simulink model "HvacMdlAirFlowDistbnFrnt"  (R2022b)
settings: Solver=FixedStepDiscrete  StartTime=0.0  StopTime=10.0  FixedStep=0.2  RelTol=1e-3
(part 2 of 2)

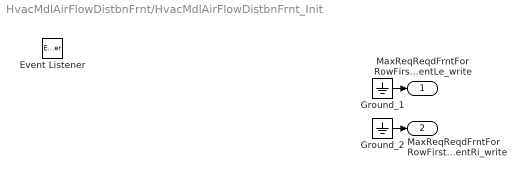
== system HvacMdlAirFlowDistbnFrnt/HvacMdlAirFlowDistbnFrnt_Init ==
  Event Listener : EventListener  EventName=reset
  Ground_1 : Ground
  Ground_2 : Ground
  MaxReqReqdFrntForRowFirstAtVent_MaxReqReqdFrntForRowFirstAtVentLe_write : Outport
  MaxReqReqdFrntForRowFirstAtVent_MaxReqReqdFrntForRowFirstAtVentRi_write : Outport  Port=2
  wires:
  Ground_1/1 -> MaxReqReqdFrntForRowFirstAtVent_MaxReqReqdFrntForRowFirstAtVentLe_write/1
  Ground_2/1 -> MaxReqReqdFrntForRowFirstAtVent_MaxReqReqdFrntForRowFirstAtVentRi_write/1

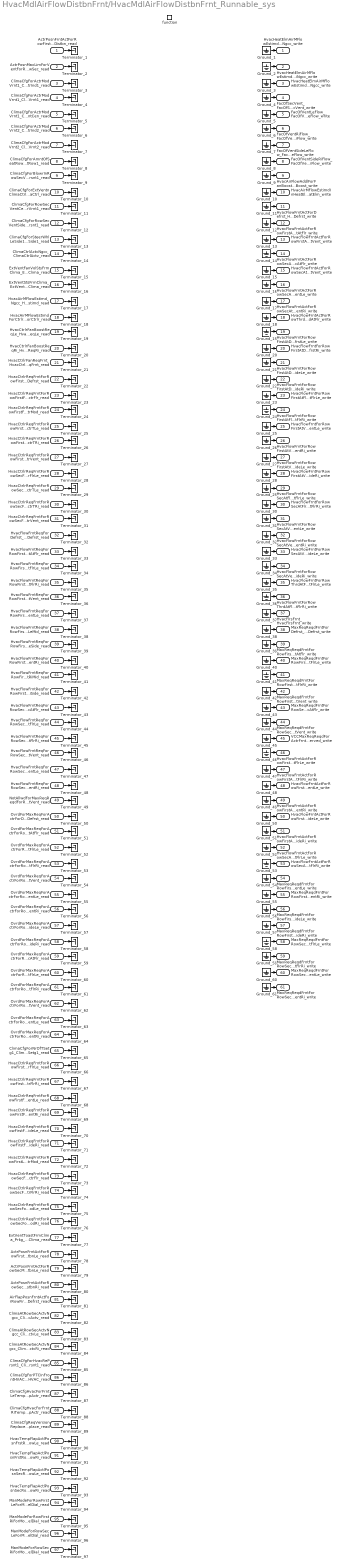
== system HvacMdlAirFlowDistbnFrnt/HvacMdlAirFlowDistbnFrnt_Runnable_sys ==
  ActrPosnFrntActForRowFirstModDistbn_ActrPosnFrntActForRowFirstModDistbn_read : Inport
  ActrPosnMaxLimForVentForRowSec_ActrPosnMaxLimForVentForRowSec_read : Inport  Port=2
  ClimaCfgForActrModVrnt1_ClimaCfgForActrInd1_read : Inport  Port=3
  ClimaCfgForActrModVrnt1_ClimaCfgForActrModVrnt1_read : Inport  Port=4
  ClimaCfgForActrModVrnt1_ClimaCfgForActrModVrntCen_read : Inport  Port=5
  ClimaCfgForActrModVrnt2_ClimaCfgForActrInd2_read : Inport  Port=6
  ClimaCfgForActrModVrnt2_ClimaCfgForActrModVrnt2_read : Inport  Port=7
  ClimaCfgForAmntOfSeatRow1_ClimaCfgForAmntOfSeatRow1_read : Inport  Port=8
  ClimaCfgForBlowrInRowSecVentSidePrsnt1_ClimaCfgForBlowrInRowSecVentSidePrsnt1_read : Inport  Port=9
  ClimaCfgForExtVentnClimaCtrl_ClimaCfgForExtVentnClimaCtrl_read : Inport  Port=10
  ClimaCfgForRowSecVentCentrVrnt1_ClimaCfgForRowSecVentCentrVrnt1_read : Inport  Port=11
  ClimaCfgForRowSecVentSidePrsnt1_ClimaCfgForRowSecVentSidePrsnt1_read : Inport  Port=12
  ClimaCfgForSteerWhlLeSide1_ClimaCfgForSteerWhlLeSide1_read : Inport  Port=13
  ClimaCtrlActvNgcc_ClimaCtrlActv_read : Inport  Port=14
  ExtVentFanVolStsFrmClima_ExtVentFanVolStsFrmClima_read : Inport  Port=15
  ExtVentStsFrmClima_ExtVentStsFrmClima_read : Inport  Port=16
  HvacAirMFlowEstimd_Ngcc_HvacAirMFlowEstimd_read : Inport  Port=17
  HvacAirMFlowEstimdForCtrlr_HvacAirMFlowEstimdForCtrlr_read : Inport  Port=18
  HvacCtrlrFanBoostReqLe_HvacCtrlrFanBoostReqLe_read : Inport  Port=19
  HvacCtrlrFanBoostReqRi_HvacCtrlrFanBoostReqRi_read : Inport  Port=20
  HvacCtrlrFanReqFrnt_HvacCtrlrFanReqFrnt_read : Inport  Port=21
  HvacCtrlrReqFrntForRowFirstForActrDefrst_HvacCtrlrReqFrntForRowFirstForActrDefrst_read : Inport  Port=22
  HvacCtrlrReqFrntForRowFirstForActrFlr_HvacCtrlrReqFrntForRowFirstForActrFlr_read : Inport  Port=23
  HvacCtrlrReqFrntForRowFirstForActrMod_HvacCtrlrReqFrntForRowFirstForActrMod_read : Inport  Port=24
  HvacCtrlrReqFrntForRowFirstForActrTLe_HvacCtrlrReqFrntForRowFirstForActrTLe_read : Inport  Port=25
  HvacCtrlrReqFrntForRowFirstForActrTRi_HvacCtrlrReqFrntForRowFirstForActrTRi_read : Inport  Port=26
  HvacCtrlrReqFrntForRowFirstForActrVent_HvacCtrlrReqFrntForRowFirstForActrVent_read : Inport  Port=27
  HvacCtrlrReqFrntForRowSecForActrFlr_HvacCtrlrReqFrntForRowSecForActrFlrLe_read : Inport  Port=28
  HvacCtrlrReqFrntForRowSecForActrTLe_HvacCtrlrReqFrntForRowSecForActrTLe_read : Inport  Port=29
  HvacCtrlrReqFrntForRowSecForActrTRi_HvacCtrlrReqFrntForRowSecForActrTRi_read : Inport  Port=30
  HvacCtrlrReqFrntForRowSecForActrVent_HvacCtrlrReqFrntForRowSecForActrVent_read : Inport  Port=31
  HvacFlowFrntReqForDefrst_HvacFlowFrntReqForDefrst_read : Inport  Port=32
  HvacFlowFrntReqForRowFirstAtFlr_HvacFlowFrntReqForRowFirstAtFlr_read : Inport  Port=33
  HvacFlowFrntReqForRowFirstAtFlr_HvacFlowFrntReqForRowFirstAtFlrLe_read : Inport  Port=34
  HvacFlowFrntReqForRowFirstAtFlr_HvacFlowFrntReqForRowFirstAtFlrRi_read : Inport  Port=35
  HvacFlowFrntReqForRowFirstAtVent_HvacFlowFrntReqForRowFirstAtVent_read : Inport  Port=36
  HvacFlowFrntReqForRowFirstAtVent_HvacFlowFrntReqForRowFirstAtVentLe_read : Inport  Port=37
  HvacFlowFrntReqForRowFirstAtVent_HvacFlowFrntReqForRowFirstAtVentLeMid_read : Inport  Port=38
  HvacFlowFrntReqForRowFirstAtVent_HvacFlowFrntReqForRowFirstAtVentLeSide_read : Inport  Port=39
  HvacFlowFrntReqForRowFirstAtVent_HvacFlowFrntReqForRowFirstAtVentRi_read : Inport  Port=40
  HvacFlowFrntReqForRowFirstAtVent_HvacFlowFrntReqForRowFirstAtVentRiMid_read : Inport  Port=41
  HvacFlowFrntReqForRowFirstAtVent_HvacFlowFrntReqForRowFirstAtVentRiSide_read : Inport  Port=42
  HvacFlowFrntReqForRowSecAtFlr_HvacFlowFrntReqForRowSecAtFlr_read : Inport  Port=43
  HvacFlowFrntReqForRowSecAtFlr_HvacFlowFrntReqForRowSecAtFlrLe_read : Inport  Port=44
  HvacFlowFrntReqForRowSecAtFlr_HvacFlowFrntReqForRowSecAtFlrRi_read : Inport  Port=45
  HvacFlowFrntReqForRowSecAtVent_HvacFlowFrntReqForRowSecAtVent_read : Inport  Port=46
  HvacFlowFrntReqForRowSecAtVent_HvacFlowFrntReqForRowSecAtVentLe_read : Inport  Port=47
  HvacFlowFrntReqForRowSecAtVent_HvacFlowFrntReqForRowSecAtVentRi_read : Inport  Port=48
  NotAllwdForMaxReqReqdForRowSecAtVent_NotAllwdForMaxReqReqdForRowSecAtVent_read : Inport  Port=49
  OvrdForMaxReqForActrForDefrst_OvrdForMaxReqForActrForDefrst_read : Inport  Port=50
  OvrdForMaxReqForActrForRowFirstAtFlr_OvrdForMaxReqForActrForRowFirstAtFlr_read : Inport  Port=51
  OvrdForMaxReqForActrForRowFirstAtFlr_OvrdForMaxReqForActrForRowFirstAtFlrLe_read : Inport  Port=52
  OvrdForMaxReqForActrForRowFirstAtFlr_OvrdForMaxReqForActrForRowFirstAtFlrRi_read : Inport  Port=53
  OvrdForMaxReqForActrForRowFirstAtVent_OvrdForMaxReqForActrForRowFirstAtVent_read : Inport  Port=54
  OvrdForMaxReqForActrForRowFirstAtVent_OvrdForMaxReqForActrForRowFirstAtVentLe_read : Inport  Port=55
  OvrdForMaxReqForActrForRowFirstAtVent_OvrdForMaxReqForActrForRowFirstAtVentRi_read : Inport  Port=56
  OvrdForMaxReqForActrForRowFirstAtVent_OvrdForMaxReqForActrForRowFirstAtVentSideLe_read : Inport  Port=57
  OvrdForMaxReqForActrForRowFirstAtVent_OvrdForMaxReqForActrForRowFirstAtVentSideRi_read : Inport  Port=58
  OvrdForMaxReqForActrForRowSecAtFlr_OvrdForMaxReqForActrForRowSecAtFlr_read : Inport  Port=59
  OvrdForMaxReqForActrForRowSecAtFlr_OvrdForMaxReqForActrForRowSecAtFlrLe_read : Inport  Port=60
  OvrdForMaxReqForActrForRowSecAtFlr_OvrdForMaxReqForActrForRowSecAtFlrRi_read : Inport  Port=61
  OvrdForMaxReqForActrForRowSecAtVent_OvrdForMaxReqForActrForRowSecAtVent_read : Inport  Port=62
  OvrdForMaxReqForActrForRowSecAtVent_OvrdForMaxReqForActrForRowSecAtVentLe_read : Inport  Port=63
  OvrdForMaxReqForActrForRowSecAtVent_OvrdForMaxReqForActrForRowSecAtVentRi_read : Inport  Port=64
  ClimaCfgForNrOfTSetg1_ClimaCfgForNrOfTSetg1_read : Inport  Port=65
  HvacCtrlrReqFrntForRowFirstForActrFlr_HvacCtrlrReqFrntForRowFirstForActrFlrLe_read : Inport  Port=66
  HvacCtrlrReqFrntForRowFirstForActrFlr_HvacCtrlrReqFrntForRowFirstForActrFlrRi_read : Inport  Port=67
  HvacCtrlrReqFrntForRowFirstForActrVent_HvacCtrlrReqFrntForRowFirstForActrVentLe_read : Inport  Port=68
  HvacCtrlrReqFrntForRowFirstForActrVent_HvacCtrlrReqFrntForRowFirstForActrVentRi_read : Inport  Port=69
  HvacCtrlrReqFrntForRowFirstForActrVent_HvacCtrlrReqFrntForRowFirstForActrVentSideLe_read : Inport  Port=70
  HvacCtrlrReqFrntForRowFirstForActrVent_HvacCtrlrReqFrntForRowFirstForActrVentSideRi_read : Inport  Port=71
  HvacCtrlrReqFrntForRowFirstLeForActrMod_HvacCtrlrReqFrntForRowFirstLeForActrMod_read : Inport  Port=72
  HvacCtrlrReqFrntForRowSecForActrFlr_HvacCtrlrReqFrntForRowSecForActrFlr_read : Inport  Port=73
  HvacCtrlrReqFrntForRowSecForActrFlr_HvacCtrlrReqFrntForRowSecForActrFlrRi_read : Inport  Port=74
  HvacCtrlrReqFrntForRowSecForActrModLe_HvacCtrlrReqFrntForRowSecForActrModLe_read : Inport  Port=75
  HvacCtrlrReqFrntForRowSecForActrModRi_HvacCtrlrReqFrntForRowSecForActrModRi_read : Inport  Port=76
  ExtVentToastFrmClima_Prkg_ExtVentToastFrmClima_read : Inport  Port=77
  ActrPosnFrntActForRowFirstModDistbnLe_ActrPosnFrntActForRowFirstModDistbnLe_read : Inport  Port=78
  ActrPosnFrntActForRowSecModDistbnLe_ActrPosnFrntActForRowSecModDistbnLe_read : Inport  Port=79
  ActrPosnFrntActForRowSecModDistbnRi_ActrPosnFrntActForRowSecModDistbnRi_read : Inport  Port=80
  AirFlapPosnFrntActForRowFirstForActrDefrst_AirFlapPosnFrntActForRowFirstForActrDefrst_read : Inport  Port=81
  ClimaAtRowSecActvNgcc_ClimaAtRowSecActv_read : Inport  Port=82
  ClimaAtRowSecActvNgcc_ClimaAtRowSecActvLe_read : Inport  Port=83
  ClimaAtRowSecActvNgcc_ClimaAtRowSecActvRi_read : Inport  Port=84
  ClimaCfgForHvacRePrsnt1_ClimaCfgForHvacRePrsnt1_read : Inport  Port=85
  ClimaCfgForPTCInFrontHVAC_ClimaCfgForPTCInFrontHVAC_read : Inport  Port=86
  ClimaCfgHvacForFrntLeTempActr_ClimaCfgHvacForFrntLeTempActr_read : Inport  Port=87
  ClimaCfgHvacForFrntRiTempActr_ClimaCfgHvacForFrntRiTempActr_read : Inport  Port=88
  ClimaCfgReqVersionReplace_ClimaCfgReqVersionReplace_read : Inport  Port=89
  HvacTempFlapActlPosnFrstRowLe_HvacTempFlapActlPosnFrstRowLe_read : Inport  Port=90
  HvacTempFlapActlPosnFrstRowRi_HvacTempFlapActlPosnFrstRowRi_read : Inport  Port=91
  HvacTempFlapActlPosnSecRowLe_HvacTempFlapActlPosnSecRowLe_read : Inport  Port=92
  HvacTempFlapActlPosnSecRowRi_HvacTempFlapActlPosnSecRowRi_read : Inport  Port=93
  ManModeForRowFirstLeForModelDial_ManModeForRowFirstLeForModelDial_read : Inport  Port=94
  ManModeForRowFirstRiForModelDial_ManModeForRowFirstRiForModelDial_read : Inport  Port=95
  ManModeForRowSecLeForModelDial_ManModeForRowSecLeForModelDial_read : Inport  Port=96
  ManModeForRowSecRiForModelDial_ManModeForRowSecRiForModelDial_read : Inport  Port=97
  function : TriggerPort  TriggerType=function-call  VariantControl=(inherit)  SampleTimeType=periodic  SampleTime=-1
  Ground_{1..61} (61 alike) : Ground
  Terminator_{1..97} (97 alike) : Terminator
  HvacHeatElmAirMFlowEstimdFirstLeNgcc_HvacHeatElmAirMFlowEstimdFirstLeNgcc_write : Outport
      settings: EnsureOutportIsVirtual=on
  HvacHeatElmAirMFlowEstimdFirstRiNgcc_HvacHeatElmAirMFlowEstimdFirstRiNgcc_write : Outport  Port=2
      settings: EnsureOutportIsVirtual=on
  HvacHeatElmAirMFlowEstimdSecLeNgcc_HvacHeatElmAirMFlowEstimdSecLeNgcc_write : Outport  Port=3
      settings: EnsureOutportIsVirtual=on
  FacOfSecVent_FacOfSecVent_write : Outport  Port=4
      settings: EnsureOutportIsVirtual=on
  FacOfVentLeFlow_FacOfVentLeFlow_write : Outport  Port=5
      settings: EnsureOutportIsVirtual=on
  FacOfVentRiFlow_FacOfVentRiFlow_write : Outport  Port=6
      settings: EnsureOutportIsVirtual=on
  FacOfVentSideLeFlow_FacOfVentSideLeFlow_write : Outport  Port=7
      settings: EnsureOutportIsVirtual=on
  FacOfVentSideRiFlow_FacOfVentSideRiFlow_write : Outport  Port=8
      settings: EnsureOutportIsVirtual=on
  HvacAirFlowAddlForFanBoost_HvacAirFlowAddlForFanBoost_write : Outport  Port=9
      settings: EnsureOutportIsVirtual=on
  HvacAirMFlowEstimdInHeatElm_HvacAirMFlowEstimdInHeatElm_write : Outport  Port=10
      settings: EnsureOutportIsVirtual=on
  HvacFlowFrntActForDefrst_HvacFlowFrntActForDefrst_write : Outport  Port=11
      settings: EnsureOutportIsVirtual=on
  HvacFlowFrntActForRowFirstAtFlr_HvacFlowFrntActForRowFirstAtFlr_write : Outport  Port=12
      settings: EnsureOutportIsVirtual=on
  HvacFlowFrntActForRowFirstAtVent_HvacFlowFrntActForRowFirstAtVent_write : Outport  Port=13
      settings: EnsureOutportIsVirtual=on
  HvacFlowFrntActForRowSecAtFlr_HvacFlowFrntActForRowSecAtFlr_write : Outport  Port=14
      settings: EnsureOutportIsVirtual=on
  HvacFlowFrntActForRowSecAtVent_HvacFlowFrntActForRowSecAtVent_write : Outport  Port=15
      settings: EnsureOutportIsVirtual=on
  HvacFlowFrntActForRowSecAtVent_HvacFlowFrntActForRowSecAtVentLe_write : Outport  Port=16
      settings: EnsureOutportIsVirtual=on
  HvacFlowFrntActForRowSecAtVent_HvacFlowFrntActForRowSecAtVentRi_write : Outport  Port=17
      settings: EnsureOutportIsVirtual=on
  HvacFlowFrntActForRowThrdAtFlr_HvacFlowFrntActForRowThrdAtFlr_write : Outport  Port=18
      settings: EnsureOutportIsVirtual=on
  HvacFlowFrntForRowFirstAtDefrstLe_HvacFlowFrntForRowFirstAtDefrstLe_write : Outport  Port=19
      settings: EnsureOutportIsVirtual=on
  HvacFlowFrntForRowFirstAtDefrstRi_HvacFlowFrntForRowFirstAtDefrstRi_write : Outport  Port=20
      settings: EnsureOutportIsVirtual=on
  HvacFlowFrntForRowFirstAtDefrstSideLe_HvacFlowFrntForRowFirstAtDefrstSideLe_write : Outport  Port=21
      settings: EnsureOutportIsVirtual=on
  HvacFlowFrntForRowFirstAtDefrstSideRi_HvacFlowFrntForRowFirstAtDefrstSideRi_write : Outport  Port=22
      settings: EnsureOutportIsVirtual=on
  HvacFlowFrntForRowFirstAtFlrLe_HvacFlowFrntForRowFirstAtFlrLe_write : Outport  Port=23
      settings: EnsureOutportIsVirtual=on
  HvacFlowFrntForRowFirstAtFlrRi_HvacFlowFrntForRowFirstAtFlrRi_write : Outport  Port=24
      settings: EnsureOutportIsVirtual=on
  HvacFlowFrntForRowFirstAtVentLe_HvacFlowFrntForRowFirstAtVentLe_write : Outport  Port=25
      settings: EnsureOutportIsVirtual=on
  HvacFlowFrntForRowFirstAtVentRi_HvacFlowFrntForRowFirstAtVentRi_write : Outport  Port=26
      settings: EnsureOutportIsVirtual=on
  HvacFlowFrntForRowFirstAtVentSideLe_HvacFlowFrntForRowFirstAtVentSideLe_write : Outport  Port=27
      settings: EnsureOutportIsVirtual=on
  HvacFlowFrntForRowFirstAtVentSideRi_HvacFlowFrntForRowFirstAtVentSideRi_write : Outport  Port=28
      settings: EnsureOutportIsVirtual=on
  HvacFlowFrntForRowSecAtFlrLe_HvacFlowFrntForRowSecAtFlrLe_write : Outport  Port=29
      settings: EnsureOutportIsVirtual=on
  HvacFlowFrntForRowSecAtFlrRi_HvacFlowFrntForRowSecAtFlrRi_write : Outport  Port=30
      settings: EnsureOutportIsVirtual=on
  HvacFlowFrntForRowSecAtVentLe_HvacFlowFrntForRowSecAtVentLe_write : Outport  Port=31
      settings: EnsureOutportIsVirtual=on
  HvacFlowFrntForRowSecAtVentRi_HvacFlowFrntForRowSecAtVentRi_write : Outport  Port=32
      settings: EnsureOutportIsVirtual=on
  HvacFlowFrntForRowSecAtVentSideLe_HvacFlowFrntForRowSecAtVentSideLe_write : Outport  Port=33
      settings: EnsureOutportIsVirtual=on
  HvacFlowFrntForRowSecAtVentSideRi_HvacFlowFrntForRowSecAtVentSideRi_write : Outport  Port=34
      settings: EnsureOutportIsVirtual=on
  HvacFlowFrntForRowThrdAtFlrLe_HvacFlowFrntForRowThrdAtFlrLe_write : Outport  Port=35
      settings: EnsureOutportIsVirtual=on
  HvacFlowFrntForRowThrdAtFlrRi_HvacFlowFrntForRowThrdAtFlrRi_write : Outport  Port=36
      settings: EnsureOutportIsVirtual=on
  HvacFlrsFrnt_HvacFlrsFrnt_write : Outport  Port=37
      settings: EnsureOutportIsVirtual=on
  MaxReqReqdFrntForDefrst_MaxReqReqdFrntForDefrst_write : Outport  Port=38
      settings: EnsureOutportIsVirtual=on
  MaxReqReqdFrntForRowFirstAtFlr_MaxReqReqdFrntForRowFirstAtFlr_write : Outport  Port=39
      settings: EnsureOutportIsVirtual=on
  MaxReqReqdFrntForRowFirstAtFlr_MaxReqReqdFrntForRowFirstAtFlrLe_write : Outport  Port=40
      settings: EnsureOutportIsVirtual=on
  MaxReqReqdFrntForRowFirstAtFlr_MaxReqReqdFrntForRowFirstAtFlrRi_write : Outport  Port=41
      settings: EnsureOutportIsVirtual=on
  MaxReqReqdFrntForRowFirstAtVent_MaxReqReqdFrntForRowFirstAtVent_write : Outport  Port=42
      settings: EnsureOutportIsVirtual=on
  MaxReqReqdFrntForRowSecAtFlr_MaxReqReqdFrntForRowSecAtFlr_write : Outport  Port=43
      settings: EnsureOutportIsVirtual=on
  MaxReqReqdFrntForRowSecAtVent_MaxReqReqdFrntForRowSecAtVent_write : Outport  Port=44
      settings: EnsureOutportIsVirtual=on
  VCCMaxReqReqdForActrFrntEnumReserved_VCCMaxReqReqdForActrFrntEnumReserved_write : Outport  Port=45
      settings: EnsureOutportIsVirtual=on
  HvacFlowFrntActForRowFirstAtFlr_HvacFlowFrntActForRowFirstAtFlrLe_write : Outport  Port=46
      settings: EnsureOutportIsVirtual=on
  HvacFlowFrntActForRowFirstAtFlr_HvacFlowFrntActForRowFirstAtFlrRi_write : Outport  Port=47
      settings: EnsureOutportIsVirtual=on
  HvacFlowFrntActForRowFirstAtVent_HvacFlowFrntActForRowFirstAtVentLe_write : Outport  Port=48
      settings: EnsureOutportIsVirtual=on
  HvacFlowFrntActForRowFirstAtVent_HvacFlowFrntActForRowFirstAtVentRi_write : Outport  Port=49
      settings: EnsureOutportIsVirtual=on
  HvacFlowFrntActForRowFirstAtVent_HvacFlowFrntActForRowFirstAtVentSideLe_write : Outport  Port=50
      settings: EnsureOutportIsVirtual=on
  HvacFlowFrntActForRowFirstAtVent_HvacFlowFrntActForRowFirstAtVentSideRi_write : Outport  Port=51
      settings: EnsureOutportIsVirtual=on
  HvacFlowFrntActForRowSecAtFlr_HvacFlowFrntActForRowSecAtFlrLe_write : Outport  Port=52
      settings: EnsureOutportIsVirtual=on
  HvacFlowFrntActForRowSecAtFlr_HvacFlowFrntActForRowSecAtFlrRi_write : Outport  Port=53
      settings: EnsureOutportIsVirtual=on
  MaxReqReqdFrntForRowFirstAtVent_MaxReqReqdFrntForRowFirstAtVentLe_write : Outport  Port=54
      settings: EnsureOutportIsVirtual=on
  MaxReqReqdFrntForRowFirstAtVent_MaxReqReqdFrntForRowFirstAtVentRi_write : Outport  Port=55
      settings: EnsureOutportIsVirtual=on
  MaxReqReqdFrntForRowFirstAtVent_MaxReqReqdFrntForRowFirstAtVentSideLe_write : Outport  Port=56
      settings: EnsureOutportIsVirtual=on
  MaxReqReqdFrntForRowFirstAtVent_MaxReqReqdFrntForRowFirstAtVentSideRi_write : Outport  Port=57
      settings: EnsureOutportIsVirtual=on
  MaxReqReqdFrntForRowSecAtFlr_MaxReqReqdFrntForRowSecAtFlrLe_write : Outport  Port=58
      settings: EnsureOutportIsVirtual=on
  MaxReqReqdFrntForRowSecAtFlr_MaxReqReqdFrntForRowSecAtFlrRi_write : Outport  Port=59
      settings: EnsureOutportIsVirtual=on
  MaxReqReqdFrntForRowSecAtVent_MaxReqReqdFrntForRowSecAtVentLe_write : Outport  Port=60
      settings: EnsureOutportIsVirtual=on
  MaxReqReqdFrntForRowSecAtVent_MaxReqReqdFrntForRowSecAtVentRi_write : Outport  Port=61
      settings: EnsureOutportIsVirtual=on
  wires:
  ActrPosnFrntActForRowFirstModDistbn_ActrPosnFrntActForRowFirstModDistbn_read/1 -> Terminator_1/1
  ActrPosnMaxLimForVentForRowSec_ActrPosnMaxLimForVentForRowSec_read/1 -> Terminator_2/1
  ClimaCfgForActrModVrnt1_ClimaCfgForActrInd1_read/1 -> Terminator_3/1
  ClimaCfgForActrModVrnt1_ClimaCfgForActrModVrnt1_read/1 -> Terminator_4/1
  ClimaCfgForActrModVrnt1_ClimaCfgForActrModVrntCen_read/1 -> Terminator_5/1
  ClimaCfgForActrModVrnt2_ClimaCfgForActrInd2_read/1 -> Terminator_6/1
  ClimaCfgForActrModVrnt2_ClimaCfgForActrModVrnt2_read/1 -> Terminator_7/1
  ClimaCfgForAmntOfSeatRow1_ClimaCfgForAmntOfSeatRow1_read/1 -> Terminator_8/1
  ClimaCfgForBlowrInRowSecVentSidePrsnt1_ClimaCfgForBlowrInRowSecVentSidePrsnt1_read/1 -> Terminator_9/1
  ClimaCfgForExtVentnClimaCtrl_ClimaCfgForExtVentnClimaCtrl_read/1 -> Terminator_10/1
  ClimaCfgForRowSecVentCentrVrnt1_ClimaCfgForRowSecVentCentrVrnt1_read/1 -> Terminator_11/1
  ClimaCfgForRowSecVentSidePrsnt1_ClimaCfgForRowSecVentSidePrsnt1_read/1 -> Terminator_12/1
  ClimaCfgForSteerWhlLeSide1_ClimaCfgForSteerWhlLeSide1_read/1 -> Terminator_13/1
  ClimaCtrlActvNgcc_ClimaCtrlActv_read/1 -> Terminator_14/1
  ExtVentFanVolStsFrmClima_ExtVentFanVolStsFrmClima_read/1 -> Terminator_15/1
  ExtVentStsFrmClima_ExtVentStsFrmClima_read/1 -> Terminator_16/1
  HvacAirMFlowEstimd_Ngcc_HvacAirMFlowEstimd_read/1 -> Terminator_17/1
  HvacAirMFlowEstimdForCtrlr_HvacAirMFlowEstimdForCtrlr_read/1 -> Terminator_18/1
  HvacCtrlrFanBoostReqLe_HvacCtrlrFanBoostReqLe_read/1 -> Terminator_19/1
  HvacCtrlrFanBoostReqRi_HvacCtrlrFanBoostReqRi_read/1 -> Terminator_20/1
  HvacCtrlrFanReqFrnt_HvacCtrlrFanReqFrnt_read/1 -> Terminator_21/1
  HvacCtrlrReqFrntForRowFirstForActrDefrst_HvacCtrlrReqFrntForRowFirstForActrDefrst_read/1 -> Terminator_22/1
  HvacCtrlrReqFrntForRowFirstForActrFlr_HvacCtrlrReqFrntForRowFirstForActrFlr_read/1 -> Terminator_23/1
  HvacCtrlrReqFrntForRowFirstForActrMod_HvacCtrlrReqFrntForRowFirstForActrMod_read/1 -> Terminator_24/1
  HvacCtrlrReqFrntForRowFirstForActrTLe_HvacCtrlrReqFrntForRowFirstForActrTLe_read/1 -> Terminator_25/1
  HvacCtrlrReqFrntForRowFirstForActrTRi_HvacCtrlrReqFrntForRowFirstForActrTRi_read/1 -> Terminator_26/1
  HvacCtrlrReqFrntForRowFirstForActrVent_HvacCtrlrReqFrntForRowFirstForActrVent_read/1 -> Terminator_27/1
  HvacCtrlrReqFrntForRowSecForActrFlr_HvacCtrlrReqFrntForRowSecForActrFlrLe_read/1 -> Terminator_28/1
  HvacCtrlrReqFrntForRowSecForActrTLe_HvacCtrlrReqFrntForRowSecForActrTLe_read/1 -> Terminator_29/1
  HvacCtrlrReqFrntForRowSecForActrTRi_HvacCtrlrReqFrntForRowSecForActrTRi_read/1 -> Terminator_30/1
  HvacCtrlrReqFrntForRowSecForActrVent_HvacCtrlrReqFrntForRowSecForActrVent_read/1 -> Terminator_31/1
  HvacFlowFrntReqForDefrst_HvacFlowFrntReqForDefrst_read/1 -> Terminator_32/1
  HvacFlowFrntReqForRowFirstAtFlr_HvacFlowFrntReqForRowFirstAtFlr_read/1 -> Terminator_33/1
  HvacFlowFrntReqForRowFirstAtFlr_HvacFlowFrntReqForRowFirstAtFlrLe_read/1 -> Terminator_34/1
  HvacFlowFrntReqForRowFirstAtFlr_HvacFlowFrntReqForRowFirstAtFlrRi_read/1 -> Terminator_35/1
  HvacFlowFrntReqForRowFirstAtVent_HvacFlowFrntReqForRowFirstAtVent_read/1 -> Terminator_36/1
  HvacFlowFrntReqForRowFirstAtVent_HvacFlowFrntReqForRowFirstAtVentLe_read/1 -> Terminator_37/1
  HvacFlowFrntReqForRowFirstAtVent_HvacFlowFrntReqForRowFirstAtVentLeMid_read/1 -> Terminator_38/1
  HvacFlowFrntReqForRowFirstAtVent_HvacFlowFrntReqForRowFirstAtVentLeSide_read/1 -> Terminator_39/1
  HvacFlowFrntReqForRowFirstAtVent_HvacFlowFrntReqForRowFirstAtVentRi_read/1 -> Terminator_40/1
  HvacFlowFrntReqForRowFirstAtVent_HvacFlowFrntReqForRowFirstAtVentRiMid_read/1 -> Terminator_41/1
  HvacFlowFrntReqForRowFirstAtVent_HvacFlowFrntReqForRowFirstAtVentRiSide_read/1 -> Terminator_42/1
  HvacFlowFrntReqForRowSecAtFlr_HvacFlowFrntReqForRowSecAtFlr_read/1 -> Terminator_43/1
  HvacFlowFrntReqForRowSecAtFlr_HvacFlowFrntReqForRowSecAtFlrLe_read/1 -> Terminator_44/1
  HvacFlowFrntReqForRowSecAtFlr_HvacFlowFrntReqForRowSecAtFlrRi_read/1 -> Terminator_45/1
  HvacFlowFrntReqForRowSecAtVent_HvacFlowFrntReqForRowSecAtVent_read/1 -> Terminator_46/1
  HvacFlowFrntReqForRowSecAtVent_HvacFlowFrntReqForRowSecAtVentLe_read/1 -> Terminator_47/1
  HvacFlowFrntReqForRowSecAtVent_HvacFlowFrntReqForRowSecAtVentRi_read/1 -> Terminator_48/1
  NotAllwdForMaxReqReqdForRowSecAtVent_NotAllwdForMaxReqReqdForRowSecAtVent_read/1 -> Terminator_49/1
  OvrdForMaxReqForActrForDefrst_OvrdForMaxReqForActrForDefrst_read/1 -> Terminator_50/1
  OvrdForMaxReqForActrForRowFirstAtFlr_OvrdForMaxReqForActrForRowFirstAtFlr_read/1 -> Terminator_51/1
  OvrdForMaxReqForActrForRowFirstAtFlr_OvrdForMaxReqForActrForRowFirstAtFlrLe_read/1 -> Terminator_52/1
  OvrdForMaxReqForActrForRowFirstAtFlr_OvrdForMaxReqForActrForRowFirstAtFlrRi_read/1 -> Terminator_53/1
  OvrdForMaxReqForActrForRowFirstAtVent_OvrdForMaxReqForActrForRowFirstAtVent_read/1 -> Terminator_54/1
  OvrdForMaxReqForActrForRowFirstAtVent_OvrdForMaxReqForActrForRowFirstAtVentLe_read/1 -> Terminator_55/1
  OvrdForMaxReqForActrForRowFirstAtVent_OvrdForMaxReqForActrForRowFirstAtVentRi_read/1 -> Terminator_56/1
  OvrdForMaxReqForActrForRowFirstAtVent_OvrdForMaxReqForActrForRowFirstAtVentSideLe_read/1 -> Terminator_57/1
  OvrdForMaxReqForActrForRowFirstAtVent_OvrdForMaxReqForActrForRowFirstAtVentSideRi_read/1 -> Terminator_58/1
  OvrdForMaxReqForActrForRowSecAtFlr_OvrdForMaxReqForActrForRowSecAtFlr_read/1 -> Terminator_59/1
  OvrdForMaxReqForActrForRowSecAtFlr_OvrdForMaxReqForActrForRowSecAtFlrLe_read/1 -> Terminator_60/1
  OvrdForMaxReqForActrForRowSecAtFlr_OvrdForMaxReqForActrForRowSecAtFlrRi_read/1 -> Terminator_61/1
  OvrdForMaxReqForActrForRowSecAtVent_OvrdForMaxReqForActrForRowSecAtVent_read/1 -> Terminator_62/1
  OvrdForMaxReqForActrForRowSecAtVent_OvrdForMaxReqForActrForRowSecAtVentLe_read/1 -> Terminator_63/1
  OvrdForMaxReqForActrForRowSecAtVent_OvrdForMaxReqForActrForRowSecAtVentRi_read/1 -> Terminator_64/1
  ClimaCfgForNrOfTSetg1_ClimaCfgForNrOfTSetg1_read/1 -> Terminator_65/1
  HvacCtrlrReqFrntForRowFirstForActrFlr_HvacCtrlrReqFrntForRowFirstForActrFlrLe_read/1 -> Terminator_66/1
  HvacCtrlrReqFrntForRowFirstForActrFlr_HvacCtrlrReqFrntForRowFirstForActrFlrRi_read/1 -> Terminator_67/1
  HvacCtrlrReqFrntForRowFirstForActrVent_HvacCtrlrReqFrntForRowFirstForActrVentLe_read/1 -> Terminator_68/1
  HvacCtrlrReqFrntForRowFirstForActrVent_HvacCtrlrReqFrntForRowFirstForActrVentRi_read/1 -> Terminator_69/1
  HvacCtrlrReqFrntForRowFirstForActrVent_HvacCtrlrReqFrntForRowFirstForActrVentSideLe_read/1 -> Terminator_70/1
  HvacCtrlrReqFrntForRowFirstForActrVent_HvacCtrlrReqFrntForRowFirstForActrVentSideRi_read/1 -> Terminator_71/1
  HvacCtrlrReqFrntForRowFirstLeForActrMod_HvacCtrlrReqFrntForRowFirstLeForActrMod_read/1 -> Terminator_72/1
  HvacCtrlrReqFrntForRowSecForActrFlr_HvacCtrlrReqFrntForRowSecForActrFlr_read/1 -> Terminator_73/1
  HvacCtrlrReqFrntForRowSecForActrFlr_HvacCtrlrReqFrntForRowSecForActrFlrRi_read/1 -> Terminator_74/1
  HvacCtrlrReqFrntForRowSecForActrModLe_HvacCtrlrReqFrntForRowSecForActrModLe_read/1 -> Terminator_75/1
  HvacCtrlrReqFrntForRowSecForActrModRi_HvacCtrlrReqFrntForRowSecForActrModRi_read/1 -> Terminator_76/1
  ExtVentToastFrmClima_Prkg_ExtVentToastFrmClima_read/1 -> Terminator_77/1
  ActrPosnFrntActForRowFirstModDistbnLe_ActrPosnFrntActForRowFirstModDistbnLe_read/1 -> Terminator_78/1
  ActrPosnFrntActForRowSecModDistbnLe_ActrPosnFrntActForRowSecModDistbnLe_read/1 -> Terminator_79/1
  ActrPosnFrntActForRowSecModDistbnRi_ActrPosnFrntActForRowSecModDistbnRi_read/1 -> Terminator_80/1
  AirFlapPosnFrntActForRowFirstForActrDefrst_AirFlapPosnFrntActForRowFirstForActrDefrst_read/1 -> Terminator_81/1
  ClimaAtRowSecActvNgcc_ClimaAtRowSecActv_read/1 -> Terminator_82/1
  ClimaAtRowSecActvNgcc_ClimaAtRowSecActvLe_read/1 -> Terminator_83/1
  ClimaAtRowSecActvNgcc_ClimaAtRowSecActvRi_read/1 -> Terminator_84/1
  ClimaCfgForHvacRePrsnt1_ClimaCfgForHvacRePrsnt1_read/1 -> Terminator_85/1
  ClimaCfgForPTCInFrontHVAC_ClimaCfgForPTCInFrontHVAC_read/1 -> Terminator_86/1
  ClimaCfgHvacForFrntLeTempActr_ClimaCfgHvacForFrntLeTempActr_read/1 -> Terminator_87/1
  ClimaCfgHvacForFrntRiTempActr_ClimaCfgHvacForFrntRiTempActr_read/1 -> Terminator_88/1
  ClimaCfgReqVersionReplace_ClimaCfgReqVersionReplace_read/1 -> Terminator_89/1
  HvacTempFlapActlPosnFrstRowLe_HvacTempFlapActlPosnFrstRowLe_read/1 -> Terminator_90/1
  HvacTempFlapActlPosnFrstRowRi_HvacTempFlapActlPosnFrstRowRi_read/1 -> Terminator_91/1
  HvacTempFlapActlPosnSecRowLe_HvacTempFlapActlPosnSecRowLe_read/1 -> Terminator_92/1
  HvacTempFlapActlPosnSecRowRi_HvacTempFlapActlPosnSecRowRi_read/1 -> Terminator_93/1
  ManModeForRowFirstLeForModelDial_ManModeForRowFirstLeForModelDial_read/1 -> Terminator_94/1
  ManModeForRowFirstRiForModelDial_ManModeForRowFirstRiForModelDial_read/1 -> Terminator_95/1
  ManModeForRowSecLeForModelDial_ManModeForRowSecLeForModelDial_read/1 -> Terminator_96/1
  ManModeForRowSecRiForModelDial_ManModeForRowSecRiForModelDial_read/1 -> Terminator_97/1
  Ground_1/1 -> HvacHeatElmAirMFlowEstimdFirstLeNgcc_HvacHeatElmAirMFlowEstimdFirstLeNgcc_write/1
  Ground_2/1 -> HvacHeatElmAirMFlowEstimdFirstRiNgcc_HvacHeatElmAirMFlowEstimdFirstRiNgcc_write/1
  Ground_3/1 -> HvacHeatElmAirMFlowEstimdSecLeNgcc_HvacHeatElmAirMFlowEstimdSecLeNgcc_write/1
  Ground_4/1 -> FacOfSecVent_FacOfSecVent_write/1
  Ground_5/1 -> FacOfVentLeFlow_FacOfVentLeFlow_write/1
  Ground_6/1 -> FacOfVentRiFlow_FacOfVentRiFlow_write/1
  Ground_7/1 -> FacOfVentSideLeFlow_FacOfVentSideLeFlow_write/1
  Ground_8/1 -> FacOfVentSideRiFlow_FacOfVentSideRiFlow_write/1
  Ground_9/1 -> HvacAirFlowAddlForFanBoost_HvacAirFlowAddlForFanBoost_write/1
  Ground_10/1 -> HvacAirMFlowEstimdInHeatElm_HvacAirMFlowEstimdInHeatElm_write/1
  Ground_11/1 -> HvacFlowFrntActForDefrst_HvacFlowFrntActForDefrst_write/1
  Ground_12/1 -> HvacFlowFrntActForRowFirstAtFlr_HvacFlowFrntActForRowFirstAtFlr_write/1
  Ground_13/1 -> HvacFlowFrntActForRowFirstAtVent_HvacFlowFrntActForRowFirstAtVent_write/1
  Ground_14/1 -> HvacFlowFrntActForRowSecAtFlr_HvacFlowFrntActForRowSecAtFlr_write/1
  Ground_15/1 -> HvacFlowFrntActForRowSecAtVent_HvacFlowFrntActForRowSecAtVent_write/1
  Ground_16/1 -> HvacFlowFrntActForRowSecAtVent_HvacFlowFrntActForRowSecAtVentLe_write/1
  Ground_17/1 -> HvacFlowFrntActForRowSecAtVent_HvacFlowFrntActForRowSecAtVentRi_write/1
  Ground_18/1 -> HvacFlowFrntActForRowThrdAtFlr_HvacFlowFrntActForRowThrdAtFlr_write/1
  Ground_19/1 -> HvacFlowFrntForRowFirstAtDefrstLe_HvacFlowFrntForRowFirstAtDefrstLe_write/1
  Ground_20/1 -> HvacFlowFrntForRowFirstAtDefrstRi_HvacFlowFrntForRowFirstAtDefrstRi_write/1
  Ground_21/1 -> HvacFlowFrntForRowFirstAtDefrstSideLe_HvacFlowFrntForRowFirstAtDefrstSideLe_write/1
  Ground_22/1 -> HvacFlowFrntForRowFirstAtDefrstSideRi_HvacFlowFrntForRowFirstAtDefrstSideRi_write/1
  Ground_23/1 -> HvacFlowFrntForRowFirstAtFlrLe_HvacFlowFrntForRowFirstAtFlrLe_write/1
  Ground_24/1 -> HvacFlowFrntForRowFirstAtFlrRi_HvacFlowFrntForRowFirstAtFlrRi_write/1
  Ground_25/1 -> HvacFlowFrntForRowFirstAtVentLe_HvacFlowFrntForRowFirstAtVentLe_write/1
  Ground_26/1 -> HvacFlowFrntForRowFirstAtVentRi_HvacFlowFrntForRowFirstAtVentRi_write/1
  Ground_27/1 -> HvacFlowFrntForRowFirstAtVentSideLe_HvacFlowFrntForRowFirstAtVentSideLe_write/1
  Ground_28/1 -> HvacFlowFrntForRowFirstAtVentSideRi_HvacFlowFrntForRowFirstAtVentSideRi_write/1
  Ground_29/1 -> HvacFlowFrntForRowSecAtFlrLe_HvacFlowFrntForRowSecAtFlrLe_write/1
  Ground_30/1 -> HvacFlowFrntForRowSecAtFlrRi_HvacFlowFrntForRowSecAtFlrRi_write/1
  Ground_31/1 -> HvacFlowFrntForRowSecAtVentLe_HvacFlowFrntForRowSecAtVentLe_write/1
  Ground_32/1 -> HvacFlowFrntForRowSecAtVentRi_HvacFlowFrntForRowSecAtVentRi_write/1
  Ground_33/1 -> HvacFlowFrntForRowSecAtVentSideLe_HvacFlowFrntForRowSecAtVentSideLe_write/1
  Ground_34/1 -> HvacFlowFrntForRowSecAtVentSideRi_HvacFlowFrntForRowSecAtVentSideRi_write/1
  Ground_35/1 -> HvacFlowFrntForRowThrdAtFlrLe_HvacFlowFrntForRowThrdAtFlrLe_write/1
  Ground_36/1 -> HvacFlowFrntForRowThrdAtFlrRi_HvacFlowFrntForRowThrdAtFlrRi_write/1
  Ground_37/1 -> HvacFlrsFrnt_HvacFlrsFrnt_write/1
  Ground_38/1 -> MaxReqReqdFrntForDefrst_MaxReqReqdFrntForDefrst_write/1
  Ground_39/1 -> MaxReqReqdFrntForRowFirstAtFlr_MaxReqReqdFrntForRowFirstAtFlr_write/1
  Ground_40/1 -> MaxReqReqdFrntForRowFirstAtFlr_MaxReqReqdFrntForRowFirstAtFlrLe_write/1
  Ground_41/1 -> MaxReqReqdFrntForRowFirstAtFlr_MaxReqReqdFrntForRowFirstAtFlrRi_write/1
  Ground_42/1 -> MaxReqReqdFrntForRowFirstAtVent_MaxReqReqdFrntForRowFirstAtVent_write/1
  Ground_43/1 -> MaxReqReqdFrntForRowSecAtFlr_MaxReqReqdFrntForRowSecAtFlr_write/1
  Ground_44/1 -> MaxReqReqdFrntForRowSecAtVent_MaxReqReqdFrntForRowSecAtVent_write/1
  Ground_45/1 -> VCCMaxReqReqdForActrFrntEnumReserved_VCCMaxReqReqdForActrFrntEnumReserved_write/1
  Ground_46/1 -> HvacFlowFrntActForRowFirstAtFlr_HvacFlowFrntActForRowFirstAtFlrLe_write/1
  Ground_47/1 -> HvacFlowFrntActForRowFirstAtFlr_HvacFlowFrntActForRowFirstAtFlrRi_write/1
  Ground_48/1 -> HvacFlowFrntActForRowFirstAtVent_HvacFlowFrntActForRowFirstAtVentLe_write/1
  Ground_49/1 -> HvacFlowFrntActForRowFirstAtVent_HvacFlowFrntActForRowFirstAtVentRi_write/1
  Ground_50/1 -> HvacFlowFrntActForRowFirstAtVent_HvacFlowFrntActForRowFirstAtVentSideLe_write/1
  Ground_51/1 -> HvacFlowFrntActForRowFirstAtVent_HvacFlowFrntActForRowFirstAtVentSideRi_write/1
  Ground_52/1 -> HvacFlowFrntActForRowSecAtFlr_HvacFlowFrntActForRowSecAtFlrLe_write/1
  Ground_53/1 -> HvacFlowFrntActForRowSecAtFlr_HvacFlowFrntActForRowSecAtFlrRi_write/1
  Ground_54/1 -> MaxReqReqdFrntForRowFirstAtVent_MaxReqReqdFrntForRowFirstAtVentLe_write/1
  Ground_55/1 -> MaxReqReqdFrntForRowFirstAtVent_MaxReqReqdFrntForRowFirstAtVentRi_write/1
  Ground_56/1 -> MaxReqReqdFrntForRowFirstAtVent_MaxReqReqdFrntForRowFirstAtVentSideLe_write/1
  Ground_57/1 -> MaxReqReqdFrntForRowFirstAtVent_MaxReqReqdFrntForRowFirstAtVentSideRi_write/1
  Ground_58/1 -> MaxReqReqdFrntForRowSecAtFlr_MaxReqReqdFrntForRowSecAtFlrLe_write/1
  Ground_59/1 -> MaxReqReqdFrntForRowSecAtFlr_MaxReqReqdFrntForRowSecAtFlrRi_write/1
  Ground_60/1 -> MaxReqReqdFrntForRowSecAtVent_MaxReqReqdFrntForRowSecAtVentLe_write/1
  Ground_61/1 -> MaxReqReqdFrntForRowSecAtVent_MaxReqReqdFrntForRowSecAtVentRi_write/1
